simulink model "HDVSim_Hybrid_Truck"  (R2019b) (continued)
== system HDVSim_Hybrid_Truck/Electric motor ==
  note: Effective motor power
  note: Electric torque
  u_EM : Inport
  n_EM [rpm] : Inport  Port=2
  U_Bat [V] : Inport  Port=3
  Abs : Abs
  Abs1 : Abs
  Divide : Product  Inputs=*/
  Electric machine efficiency mapping : Lookup_n-D  BreakpointsForDimension1=em.efficiency.torque  BreakpointsForDimension2=em. efficiency.speed  Table=em.efficiency.characteristic_map  RndMeth=Simplest  InputSameDT=on
  Electric machine efficiency mapping2 : Lookup_n-D  BreakpointsForDimension1=em.efficiency.torque  BreakpointsForDimension2=em. efficiency.speed  Table=em.efficiency.characteristic_map  RndMeth=Simplest  InputSameDT=on
  Gain : Gain  Gain=2*pi/60
  Machine current demand : Subsystem
  Machine torque demand : Subsystem
  Math Function : Math  Operator=reciprocal  SaturateOnIntegerOverflow=on
  Product1 : Product
  Product2 : Product  Inputs=3
  Saturation : Saturate  UpperLimit=Bat.Useable_capacity / Bat.Useable_range*10  LowerLimit=-Bat.Useable_capacity / Bat.Useable_range*10
  Saturation1 : Saturate  UpperLimit=inf  LowerLimit=1
  Speed-torrque characteristics : Lookup_n-D  NumberOfTableDimensions=1  BreakpointsForDimension1=em.speed  Table=em.trq  ExtrapMethod=Clip  RndMeth=Simplest  InputSameDT=on
  Switch : Switch
  M_EM [Nm] : Outport  VectorParamsAs1DForOutWhenUnconnected=off
  I_EM_current [A] : Outport  Port=2  VectorParamsAs1DForOutWhenUnconnected=off
  tag scope: M_EM=global, n_EM=global, u_EM=global
  wires:
  Speed-torrque characteristics/1 -> Product1/1  "M_EM_max"
  u_EM/1 -> Product1/2, [u_EM]
  U_Bat [V]/1 -> Divide/2, Machine torque demand/4(U_Bat [V]), Machine current demand/4(U_Bat [V])  "U_Bat [V]"
  Machine current demand/1(I_target [A]) -> Saturation/1
  Saturation/1 -> Machine torque demand/2(I_current [A])
  Machine torque demand/1(M_EM [Nm]) -> Abs/1, Product2/3, [M_EM], M_EM [Nm]/1, Switch/2
  Gain/1 -> Product2/2
  Divide/1 -> I_EM_current [A]/1
  Math Function/1 -> Switch/1
  Electric machine efficiency mapping/1 -> Switch/3, Math Function/1  "Eta_EM"
  Switch/1 -> Product2/1
  Product2/1 -> Divide/1
  Abs/1 -> Electric machine efficiency mapping/1
  Product1/1 -> Machine current demand/2(M_EM_target [Nm]), Abs1/1  "M_EM_soll"
  n_EM [rpm]/1 -> Electric machine efficiency mapping2/2, Saturation1/1, Electric machine efficiency mapping/2, Machine torque demand/1(n_EM [rpm]), Machine current demand/1("n_EM "), [n_EM], Speed-torrque characteristics/1  "n_EM [1/min]"
  Abs1/1 -> Electric machine efficiency mapping2/1
  Electric machine efficiency mapping2/1 -> Machine current demand/3(Eff_EM), Machine torque demand/3(Eff_EM)  "ETA_EM"
  Saturation1/1 -> Gain/1

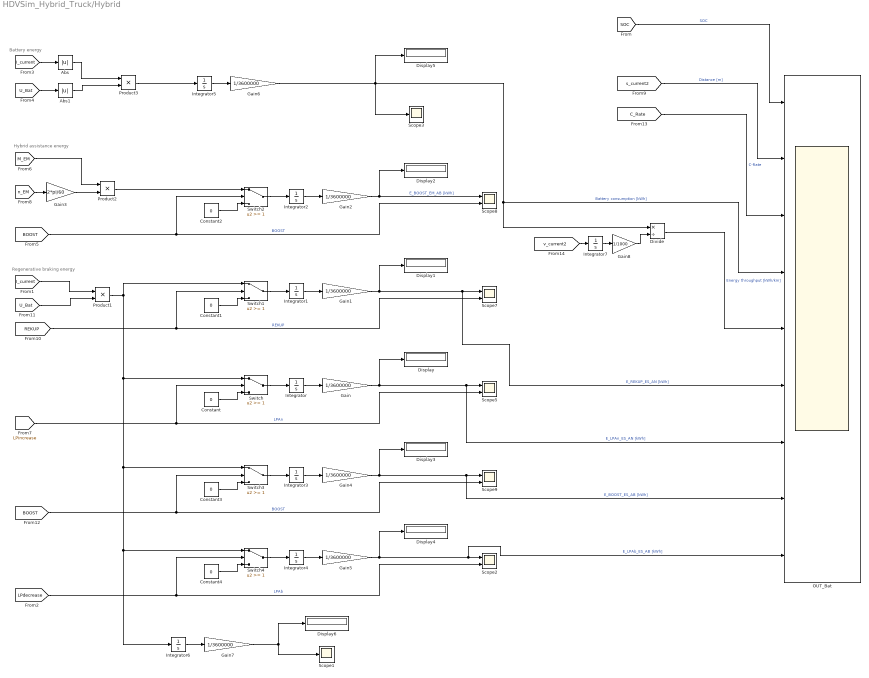
== system HDVSim_Hybrid_Truck/Hybrid ==
  note: Regenerative braking energy
  note: Hybrid assistance energy
  note: Battery energy
  Abs : Abs
  Abs1 : Abs
  Constant{,1..4} (5 alike) : Constant  Value=0
  Display{,1..6} (7 alike) : Display
  Divide : Product  Inputs=*/
  Gain{,1..2,4..7} (7 alike) : Gain  Gain=1/3600000
  Gain3 : Gain  Gain=2*pi/60
  Gain8 : Gain  Gain=1/1000
  Integrator{,1..6} (7 alike) : Integrator
  Integrator7 : Integrator  InitialCondition=0.00000001
  OUT_Bat : Scope  NumInputPorts=9
  Product1 : Product
  Product2 : Product
  Product3 : Product
  Scope1 : Scope
  Scope{2,5,7..9} (5 alike) : Scope  NumInputPorts=2
  Scope3 : Scope
  Switch{,1..4} (5 alike) : Switch  Threshold=1
  wires:
  Product1/1 -> Switch1/1, Switch/1, Switch3/1, Integrator6/1, Switch4/1
  Gain5/1 -> OUT_Bat/9, Scope2/1, Display4/1  "E_LPAb_ES_AB [kWh]"
  Constant4/1 -> Switch4/3
  Integrator4/1 -> Gain5/1
  Switch4/1 -> Integrator4/1
  [LPdecrease] -> Scope2/2, Switch4/2  "LPAb"
  Gain4/1 -> OUT_Bat/8, Scope9/1, Display3/1  "E_BOOST_ES_AB [kWh]"
  Constant3/1 -> Switch3/3
  Integrator3/1 -> Gain4/1
  Switch3/1 -> Integrator3/1
  [BOOST] -> Scope9/2, Switch3/2  "BOOST"
  Gain3/1 -> Product2/2
  [n_EM] -> Gain3/1
  Gain2/1 -> Scope8/1, Display2/1  "E_BOOST_EM_AB [kWh]"
  Constant2/1 -> Switch2/3
  Integrator2/1 -> Gain2/1
  Switch2/1 -> Integrator2/1
  [BOOST] -> Scope8/2, Switch2/2  "BOOST"
  Product2/1 -> Switch2/1
  Gain1/1 -> OUT_Bat/6, Scope7/1, Display1/1  "E_REKUP_ES_AN [kWh]"
  Constant1/1 -> Switch1/3
  Integrator1/1 -> Gain1/1
  Switch1/1 -> Integrator1/1
  [REKUP] -> Scope7/2, Switch1/2  "REKUP"
  [U_Bat] -> Product1/2
  [I_current] -> Product1/1
  Gain/1 -> OUT_Bat/7, Scope5/1, Display/1  "E_LPAn_ES_AN [kWh]"
  Constant/1 -> Switch/3
  Integrator/1 -> Gain/1
  Switch/1 -> Integrator/1
  [LPincrease] -> Scope5/2, Switch/2  "LPAn"
  [M_EM] -> Product2/1
  [SOC] -> OUT_Bat/1  "SOC"
  Gain7/1 -> Display6/1, Scope1/1
  Integrator6/1 -> Gain7/1
  [U_Bat] -> Abs1/1
  [I_current] -> Abs/1
  Product3/1 -> Integrator5/1
  Integrator5/1 -> Gain6/1
  Abs/1 -> Product3/1
  Abs1/1 -> Product3/2
  [s_current2] -> OUT_Bat/2  "Distance [m]"
  [C_Rate] -> OUT_Bat/3  "C-Rate"
  Divide/1 -> OUT_Bat/5  "Energy throughput [kWh/km]"
  [v_current2] -> Integrator7/1
  Integrator7/1 -> Gain8/1
  Gain8/1 -> Divide/2
  Gain6/1 -> Scope3/1, OUT_Bat/4, Divide/1, Display5/1  "Battery consumption [kWh]"

== system HDVSim_Hybrid_Truck/ICE engine ==
  note: Engine loading
  note: Consumption calculations
  u_ICE : Inport
  n_ICE [rpm] : Inport  Port=2
  Additional consumptions : Subsystem
  Consumption : Subsystem
  Drag torque : Lookup  InputValues=engine.drag_torque.speed  Table=engine.drag_torque.trq  LookUpMeth=Interpolation-Use End Values
  Full load curve : Lookup  InputValues=engine.full_load.speed  Table=engine.full_load.trq  LookUpMeth=Interpolation-Use End Values
  MinMax : MinMax  Inputs=2
  Product1 : Product
  Sum : Sum  Inputs=|+-
  Sum1 : Sum  Inputs=|++
  maximum engine torque due to tire slip : Subsystem
  M_ICE [Nm] : Outport  VectorParamsAs1DForOutWhenUnconnected=off
  tag scope: M_mot=global, n_mot=global
  wires:
  n_ICE [rpm]/1 -> Additional consumptions/2(n_clutch_en [rpm]), Consumption/2(n [rpm]), [n_mot], Drag torque/1, Full load curve/1
  Sum/1 -> Product1/2
  Product1/1 -> Sum1/1
  Drag torque/1 -> Sum1/2, Sum/2  "M_Drag"
  Additional consumptions/1(M_ICE_aftr_aux [Nm]) -> M_ICE [Nm]/1
  u_ICE/1 -> Product1/1
  Full load curve/1 -> MinMax/2  "M_ICE_max"
  Sum1/1 -> [M_mot], Additional consumptions/1(M_ICE_bfor_aux [Nm]), Consumption/1(M [Nm])
  maximum engine torque due to tire slip/1(M_mot_max_traction [Nm]) -> MinMax/1
  MinMax/1 -> Sum/1

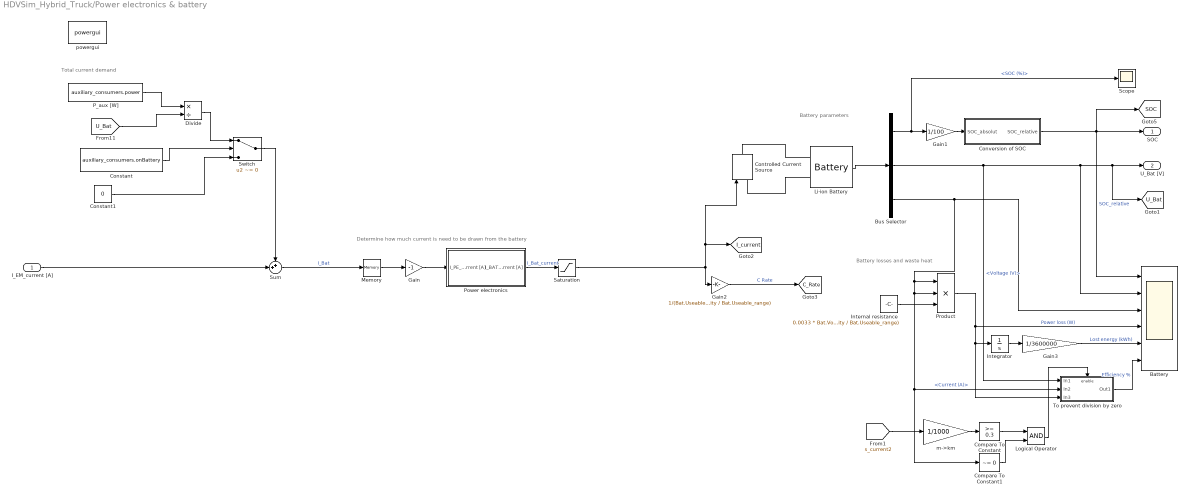
== system HDVSim_Hybrid_Truck/Power electronics & battery ==
  note: Battery parameters
  note: Battery losses and waste heat
  note: Total current demand
  note: Determine how much current is need to be drawn from the battery
  I_EM_current [A] : Inport
  Battery : Scope  NumInputPorts=6
  Bus Selector : BusSelector  OutputSignals=SOC (%),Voltage (V),Current (A)
  Compare To Constant : simulink/Logic and Bit Operations/Compare To Constant  relop=>=  const=0.3  OutDataTypeStr=uint8  ZeroCross=off
  Compare To Constant1 : simulink/Logic and Bit Operations/Compare To Constant  relop=~=  const=0  OutDataTypeStr=uint8  ZeroCross=off
  Constant : Constant  Value=auxiliary_consumers.onBattery
  Constant1 : Constant  Value=0
  Controlled Current Source : powerlib/Electrical Sources/Controlled Current Source  Initialize=off  Source_Type=DC  Amplitude=0  Phase=0  Frequency=0  Measurements=None
      settings: SourceProductName=Simscape Electrical
  Conversion of SOC : Subsystem
  Divide : Product  Inputs=*/
  Gain : Gain  Gain=-1
  Gain1 : Gain  Gain=1/100
  Gain2 : Gain  Gain=1/(Bat.Useable_capacity / Bat.Useable_range)
  Gain3 : Gain  Gain=1/3600000
  Integrator : Integrator
  Internal resistance : Constant  Value=0.0033 * Bat.Voltage / (Bat.Useable_capacity / Bat.Useable_range)
  Li-ion Battery : electricdrivelib/Extra Sources/Battery  BatType=Lithium-Ion  ShowTempParam=off  ThermalPreset=no  ShowAgeParam=off  NomV=Bat.Voltage  NomQ=Bat.Useable_capacity / Bat.Useable_range  SOC=(Bat.SOC_start *Bat.Useable_range+(0.5-Bat.Useable_range/2))* 100  Batt_Tr=30  PresetModel=off  MaxQ=Bat.Useable_capacity / Bat.Useable_range *8077/7500  MinV=487.5  FullV=Bat.Voltage * 1.178  Dis_rate=Bat.Useable_capacity / Bat.Useable_range * 1  R=0.0033 * Bat.Voltage / (Bat.Useable_capacity / Bat.Useable_range)  Normal_OP=Bat.Useable_capacity / Bat.Useable_range * 0.962  expZone="[Bat.Voltage*4339/4000     Bat.Useable_capacity / Bat.Useable_range/5]"  current=[5 25 50 250]  Units=Ampere-hour  Tci=20  NomT=20  T2=-30  MaxQ2=Bat.Useable_capacity / Bat.Useable_range *8077/7500  FullV2=Bat.Voltage * 1.178  Normal_OP2=487.5  expZone2="[Bat.Voltage*4339/4000     Bat.Useable_capacity / Bat.Useable_range/5]"  Rca=0.6  ti=1000  Dp=0  Neq0=0  Tsim=1e6  Ta1=25  Qeol=5.4*0.9  Reol=0.013333*1.2  Char_age=[2.3478, 3]  Dis_age=[2.3478, 10]  N1=1500  N2=10500  N3=1000  N4=1400  Ta2=45  N5=950
      settings: SourceProductName=Simscape Electrical
  Logical Operator : Logic  AllPortsSameDT=off  OutDataTypeStr=boolean
  Memory : Memory
  P_aux [W] : Constant  Value=auxiliary_consumers.power
  Power electronics : Subsystem
  Product : Product  Inputs=3
  Saturation : Saturate  UpperLimit=450  LowerLimit=-450
  Scope : Scope
  Sum : Sum  Inputs=++|
  Switch : Switch  Criteria=u2 ~= 0
  To prevent division by zero : Subsystem
  m->km : Gain  Gain=1/1000
  powergui : powerlib/powergui  Priority=1  SimulationMode=Discrete  SampleTime=sample_time  frequency=60  Iterations=50  frequencyindice=0  Pbase=100e6  ErrMax=1e-4  UnitsV=kV  UnitsW=MW  buscounter=0  FunctionMessages=off  echomessages=off  EnableUseOfTLC=off  CurrentSourceSwitches=off  DisableSnubberDevices=off  DisableRonSwitches=off  DisableVfSwitches=off  DisplayEquations=off  AutomaticDiscreteSolvers=off  SolverType=Tustin  Interpol=off  ExternalGateDelay=off  methode=off  Ts=100  x0status=blocks  SPID=off  SwTol=0  HookPort=off  NonlinearTolerance=1e-4  nMaxIteration=100  ContinueOnMaxIteration=off  RestoreLinks=warning  ResistiveCurrentMeasurement=off  Frange=[0:2:500]  Ylog=off  Xlog=on  ShowGrid=off  save=off  variable=ZData  structure=0  ZoomFFT=off  StartTime=0.0  cycles=1  DisplayStyle=1  fundamental=60  FreqAxis=off  MaxFrequency=1000  frequencyindicesteady=1  RmsSteady=1  display=off
      settings: SourceProductName=Simscape Electrical
  SOC : Outport  VectorParamsAs1DForOutWhenUnconnected=off
  U_Bat [V] : Outport  Port=2  VectorParamsAs1DForOutWhenUnconnected=off
  tag scope: C_Rate=global, I_current=global, SOC=global, U_Bat=global
  wires:
  Li-ion Battery/1 -> Bus Selector/1
  Bus Selector/1 -> Scope/1, Gain1/1  "<SOC (%)>"
  Bus Selector/2 -> To prevent division by zero/1(In1), U_Bat [V]/1, [U_Bat], Battery/2  "<Voltage (V)>"
  Gain1/1 -> Conversion of SOC/1(SOC_absolut)
  Conversion of SOC/1(SOC_relative) -> SOC/1, [SOC], Battery/1  "SOC_relative"
  I_EM_current [A]/1 -> Sum/2
  Power electronics/1(I_BAT_current [A]) -> Saturation/1  "I_Bat_current"
  Gain2/1 -> [C_Rate]  "C Rate"
  [U_Bat] -> Divide/2
  P_aux [W]/1 -> Divide/1
  Constant/1 -> Switch/2
  Divide/1 -> Switch/1
  Constant1/1 -> Switch/3
  Switch/1 -> Sum/1
  Bus Selector/3 -> Product/1, To prevent division by zero/2(In2), Compare To Constant1/1, Product/2, Battery/3  "<Current (A)>"
  Internal resistance/1 -> Product/3
  Product/1 -> Battery/4, To prevent division by zero/3(In3), Integrator/1  "Power loss (W)"
  Integrator/1 -> Gain3/1
  Gain3/1 -> Battery/5  "Lost energy (kWh)"
  [s_current2] -> m->km/1
  m->km/1 -> Compare To Constant/1
  To prevent division by zero/1(Out1) -> Battery/6  "Efficiency %"
  Compare To Constant/1 -> Logical Operator/1
  Logical Operator/1 -> To prevent division by zero/Enable
  Compare To Constant1/1 -> Logical Operator/2
  Saturation/1 -> Controlled Current Source/1, [I_current], Gain2/1
  Gain/1 -> Power electronics/1(I_PE_current [A])
  Sum/1 -> Memory/1  "I_Bat"
  Memory/1 -> Gain/1
  connections:
  Li-ion Battery/LConn1 -- Controlled Current Source/RConn1
  Li-ion Battery/LConn2 -- Controlled Current Source/LConn1

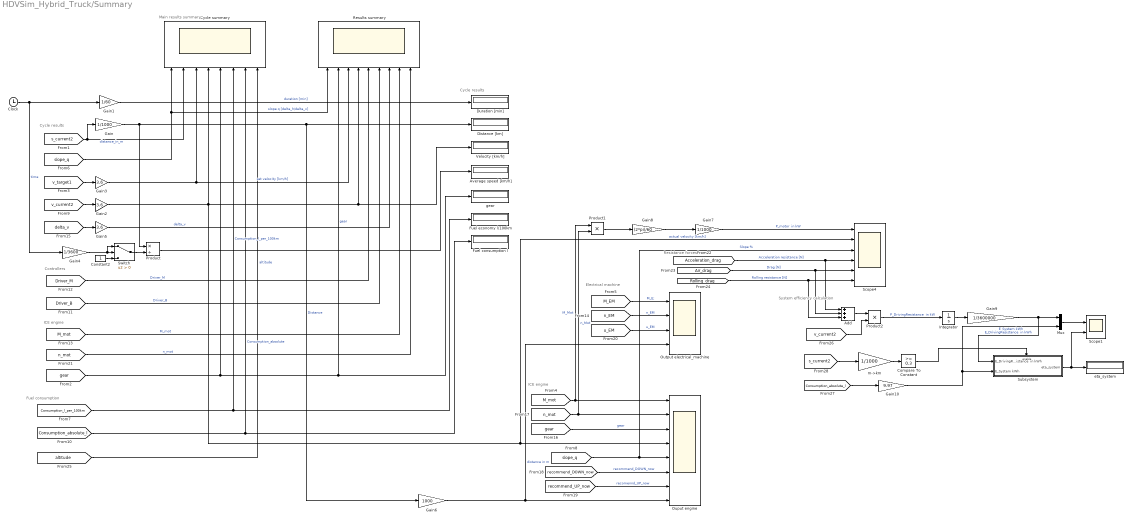
== system HDVSim_Hybrid_Truck/Summary ==
  note: Resistance forces
  note: Electrical machine
  note: ICE engine
  note: Main results summary
  note: Cycle results
  note: Fuel consumption
  note: ICE engine
  note: Controllers
  note: Cycle results
  note: System efficiency calculation
  Add : Sum  Inputs=+++
  Average speed [km/h] : Display
  Clock : Clock
  Compare To Constant : simulink/Logic and Bit Operations/Compare To Constant  relop=>=  const=0.3  OutDataTypeStr=uint8  ZeroCross=off
  Constant2 : Constant
  Cycle summary : Scope  NumInputPorts=8
  Distance [km] : Display
  Duration [min] : Display
  Fuel consumption l : Display
  Fuel economy l/100km : Display
  Gain : Gain  Gain=1/1000
  Gain1 : Gain  Gain=1/60
  Gain10 : Gain  Gain=9.97
  Gain2 : Gain  Gain=3.6
  Gain3 : Gain  Gain=3.6
  Gain4 : Gain  Gain=1/3600
  Gain5 : Gain  Gain=3.6
  Gain6 : Gain  Gain=1000
  Gain7 : Gain  Gain=1/1000
  Gain8 : Gain  Gain=(2*pi)/60
  Gain9 : Gain  Gain=1/3600000
  Integrator : Integrator
  Mux : Mux  Inputs=2
  Ouput engine : Scope  NumInputPorts=8
  Output electrical_machine : Scope  NumInputPorts=4
  Product : Product  Inputs=*/  RndMeth=Zero
  Product1 : Product
  Product2 : Product
  Results summary : Scope  NumInputPorts=9
  Scope1 : Scope  NumInputPorts=2
  Scope4 : Scope  NumInputPorts=6
  Subsystem : Subsystem
  Switch : Switch  Criteria=u2 > Threshold
  Velocity [km/h] : Display
  eta_system : Display
  gear : Display
  m->km : Gain  Gain=1/1000
  wires:
  [v_current2] -> Gain2/1
  [s_current2] -> Cycle summary/2, Gain/1  "distance_in_m"
  Gain/1 -> Distance [km]/1, Gain6/1, Product/1  "Distance"
  Clock/1 -> Gain1/1, Gain4/1  "time"
  Gain1/1 -> Duration [min]/1  "duration [min]"
  Gain2/1 -> Ouput engine/4, Scope4/2, Results summary/4, Velocity [km/h]/1, Cycle summary/4  "actual velocity [km/h]"
  [gear] -> gear/1, Results summary/2, Cycle summary/5  "gear"
  [v_target1] -> Gain3/1
  [slope_q] -> Results summary/1, Cycle summary/1  "slope q [delta_h/delta_x]"
  [Consumption_l_per_100km] -> Cycle summary/6, Fuel economy l/100km/1  "Consumption_l_per_100km"
  Gain3/1 -> Cycle summary/3, Results summary/3  "set velocity [km/h]"
  Product/1 -> Average speed [km/h]/1
  [Consumption_absolute_l] -> Cycle summary/7, Fuel consumption l/1  "Consumption_absolute"
  [Driver_M] -> Results summary/5  "Driver_M"
  [Driver_B] -> Results summary/6  "Driver_B"
  [M_mot] -> Results summary/8  "M_mot"
  [delta_v] -> Gain5/1
  [M_mot] -> Ouput engine/1, Product1/1  "M_Mot"
  [n_mot] -> Ouput engine/2, Product1/2  "n_Mot"
  [gear] -> Ouput engine/3  "gear"
  [recommend_DOWN_now] -> Ouput engine/6  "recommend_DOWN_now"
  [recommend_UP_now] -> Ouput engine/7  "recomennd_UP_now"
  [slope_q] -> Ouput engine/5, Scope4/3  "Slope %"
  Gain5/1 -> Results summary/7  "delta_v"
  [n_mot] -> Results summary/9  "n_mot"
  [M_EM] -> Output electrical_machine/1  "M_E;"
  [n_EM] -> Output electrical_machine/2  "n_EM"
  [u_EM] -> Output electrical_machine/3  "u_EM"
  Product1/1 -> Gain8/1
  Gain7/1 -> Scope4/1  "P_motor  in kW"
  Gain4/1 -> Switch/1, Switch/2
  Constant2/1 -> Switch/3
  Switch/1 -> Product/2
  [altitude] -> Cycle summary/8  "altitude"
  Gain6/1 -> Output electrical_machine/4, Ouput engine/8  "distance in m"
  Gain8/1 -> Gain7/1
  Subsystem/1(eta_system) -> eta_system/1, Scope1/2
  Mux/1 -> Scope1/1
  [v_current2] -> Product2/2
  [s_current2] -> m->km/1
  m->km/1 -> Compare To Constant/1
  Gain10/1 -> Mux/2, Subsystem/2(E_System kWh)  "E_System kWh"
  Gain9/1 -> Subsystem/1(E_DrivingResistance  in kWh), Mux/1  "E_DrivingResistance  in kWh"
  Integrator/1 -> Gain9/1
  [Consumption_absolute_l] -> Gain10/1
  Add/1 -> Product2/1
  Product2/1 -> Integrator/1  "P_DrivingResistance  in kW"
  Compare To Constant/1 -> Subsystem/Enable
  [Acceleration_drag] -> Add/1, Scope4/4  "Acceleration resistance [N]"
  [Air_drag] -> Add/2, Scope4/5  "Drag [N]"
  [Rolling_drag] -> Add/3, Scope4/6  "Rolling resistance [N]"

== system HDVSim_Hybrid_Truck/Tires ==
  M_tire [Nm] : Inport
  v_current [m/s] : Inport  Port=2
  F=M/r : Product  Inputs=*/
  Tire radius : Constant  Value=tires.radius
  "phi. -> n[1/min]" : Gain  Gain=60/2/pi
  phi. = v/r : Product  Inputs=/*
  n_tire [rpm] : Outport  VectorParamsAs1DForOutWhenUnconnected=off
  F_x [N] : Outport  Port=2  VectorParamsAs1DForOutWhenUnconnected=off
  wires:
  phi. = v/r/1 -> "phi. -> n[1/min]"/1
  "phi. -> n[1/min]"/1 -> n_tire [rpm]/1
  Tire radius/1 -> F=M/r/2, phi. = v/r/1
  v_current [m/s]/1 -> phi. = v/r/2
  M_tire [Nm]/1 -> F=M/r/1
  F=M/r/1 -> F_x [N]/1

== system HDVSim_Hybrid_Truck/Transmission ==
  note: Transmission torque multiplication through the different gears
  M_EM [Nm] : Inport
  M_clutch_dis [Nm] : Inport  Port=2
  n_Gearbox [rpm] : Inport  Port=3
  alpha : Inport  Port=4
  Determine when shifting occurs : Subsystem  TreatAsAtomicUnit=on
  Memory1 : Memory
  Number of gears : Constant  Value=length(transmission.ratios)
  Saturation : Saturate  UpperLimit=4000  LowerLimit=0
  Shifting strategy1 : Stateflow chart  TreatAsAtomicUnit=on
  Sum : Sum
  Torque flow through the transmission : Subsystem
  ZKU : Subsystem
  init_gear : Constant  Value=transmission.init_gear
  n_clutch_dis [rpm] : Outport  VectorParamsAs1DForOutWhenUnconnected=off
  M_Gearbox [Nm] : Outport  Port=2  VectorParamsAs1DForOutWhenUnconnected=off
  tag scope: gear=global, i_gearbox=global, recommend_DOWN_now=global, recommend_UP_now=global
  wires:
  n_Gearbox [rpm]/1 -> Torque flow through the transmission/2(n_gearbox_out [1/min]), Determine when shifting occurs/2(n_Gear_out [1/min])
  Torque flow through the transmission/2(M_gearbox_out [Nm]) -> [M_Gear_out], M_Gearbox [Nm]/1
  Torque flow through the transmission/1(n_gearbox_in [1/min]) -> Saturation/1
  init_gear/1 -> Shifting strategy1/1(init_gear)
  Determine when shifting occurs/27(check_torque_reseve_2lower) -> Shifting strategy1/29(check_torque_reserve_2lower)
  Determine when shifting occurs/26(check_torque_reseve_2higher) -> Shifting strategy1/28(check_torque_reserve_2higher)
  Determine when shifting occurs/1(reccommend_down_2higher) -> Shifting strategy1/3(recommend_down_2higher)
  Determine when shifting occurs/2(reccommend_down_1higher) -> Shifting strategy1/4(recommend_down_1higher)
  Determine when shifting occurs/3(reccommend_down_now) -> Shifting strategy1/5(recommend_down_now), [recommend_DOWN_now]
  Determine when shifting occurs/4(reccommend_down_1lower) -> Shifting strategy1/6(recommend_down_1lower)
  Determine when shifting occurs/5(reccommend_down_2lower) -> Shifting strategy1/7(recommend_down_2llower)
  Determine when shifting occurs/6(reccommend_up_2higher) -> Shifting strategy1/8(recommend_up_2higher)
  Determine when shifting occurs/7(reccommend_up_1higher) -> Shifting strategy1/9(recommend_up_1higher)
  Determine when shifting occurs/8(reccommend_up_now) -> Shifting strategy1/10(recommend_up_now), [recommend_UP_now]
  Determine when shifting occurs/9(reccommend_up_1lower) -> Shifting strategy1/11(recommend_up_1lower)
  Determine when shifting occurs/10(reccommend_up_2lower) -> Shifting strategy1/12(recommend_up_2lower)
  Determine when shifting occurs/11(check_n_min_2higher) -> Shifting strategy1/13(check_n_min_2higher)
  Determine when shifting occurs/12(check_n_min_1higher) -> Shifting strategy1/14(check_n_min_1higher)
  Determine when shifting occurs/13(check_n_min_now) -> Shifting strategy1/15(check_n_min_now)
  Determine when shifting occurs/14(check_n_min_1lower) -> Shifting strategy1/16(check_n_min_1lower)
  Determine when shifting occurs/15(check_n_min_2lower) -> Shifting strategy1/17(check_n_min_2lower)
  Determine when shifting occurs/16(check_n_max_2higher) -> Shifting strategy1/18(check_n_max_2higher)
  Determine when shifting occurs/17(check_n_max_1higher) -> Shifting strategy1/19(check_n_max_1higher)
  Determine when shifting occurs/18(check_n_max_now) -> Shifting strategy1/20(check_n_max_now)
  Determine when shifting occurs/19(check_n_max_1lower) -> Shifting strategy1/21(check_n_max_1lower)
  Determine when shifting occurs/20(check_n_max_2lower) -> Shifting strategy1/22(check_n_max_2lower)
  Determine when shifting occurs/21(check_M_max_2higher) -> Shifting strategy1/23(check_M_max_2higher)  "check_M_max_2higher"
  Determine when shifting occurs/22(check_M_max_1higher) -> Shifting strategy1/24(check_M_max_1higher)  "check_M_max_1higher"
  Determine when shifting occurs/23(check_M_max_now) -> Shifting strategy1/25(check_M_max_now)  "check_M_max_now"
  Determine when shifting occurs/24(check_M_max_1lower) -> Shifting strategy1/26(check_M_max_1lower)  "check_M_max_1lower"
  Determine when shifting occurs/25(check_M_max_2lower) -> Shifting strategy1/27(check_M_max_2lower)  "check_M_max_2lower"
  Memory1/1 -> [Gear], ZKU/1(Gear), Torque flow through the transmission/3(Gear), [gear]
  M_clutch_dis [Nm]/1 -> Sum/2
  Sum/1 -> Torque flow through the transmission/1(M_gearbox_in [Nm])  "M_gearbox_in [Nm]"
  Torque flow through the transmission/3(i_gearbox) -> [i_gearbox]
  Saturation/1 -> n_clutch_dis [rpm]/1
  M_EM [Nm]/1 -> Sum/1, Determine when shifting occurs/4(M_EM [Nm])
  Number of gears/1 -> Shifting strategy1/2(z)
  alpha/1 -> Determine when shifting occurs/5(alpha)
  [gear] -> Memory1/1
  Shifting strategy1/1(gear) -> [gear]
  [Gear] -> Determine when shifting occurs/1(Gear)
  [M_Gear_out] -> Determine when shifting occurs/3(M_Gear_out [Nm])

== system HDVSim_Hybrid_Truck/Vehicle ==
  note: Net tractive force applied on the vehicle
  note: Vehicle acceleration
  F_x [N] : Inport
  u_KB : Inport  Port=2
  alpha [rad] : Inport  Port=3
  Add : Sum  Inputs=-+-
  Breaking force [N] : Product  RndMeth=Zero
  Cargo weight : Constant  Value=vehicle.payload
  Driving resistances without acceleration : Subsystem
  F_brake_max : Constant  Value=vehicle.F_brake_max
  Integrator : Integrator
  Integrator1 : Integrator  InitialCondition=cycle.speed_init  LowerSaturationLimit=0
  Product : Product  Inputs=3
  Product2 : Product  Inputs=*/  RndMeth=Zero
  Product3 : Product  Inputs=**  RndMeth=Zero
  Saturation : Saturate  UpperLimit=15  LowerLimit=-15
  Sum1 : Sum  Inputs=|++
  Sum2 : Sum  Inputs=|++
  Vehicle weight : Constant  Value=vehicle.mass
  v_current [m/s] : Outport  VectorParamsAs1DForOutWhenUnconnected=off
  s_current [m] : Outport  Port=2  VectorParamsAs1DForOutWhenUnconnected=off
  tag scope: Acceleration_drag=global, s_current2=global, v_current2=global
  wires:
  alpha [rad]/1 -> Driving resistances without acceleration/1(alpha [rad])
  Add/1 -> Product2/1
  Driving resistances without acceleration/1(F_W [N]) -> Add/3
  F_x [N]/1 -> Add/2  "F_x"
  F_brake_max/1 -> Breaking force [N]/2
  Integrator/1 -> [s_current2], s_current [m]/1  "s_ist"
  Cargo weight/1 -> Sum1/2, Sum2/2
  Sum1/1 -> Product2/2
  Vehicle weight/1 -> Sum2/1, Product3/2
  Product3/1 -> Sum1/1
  Integrator1/1 -> Driving resistances without acceleration/2(v [m/s]), v_current [m/s]/1, Integrator/1, [v_current2]  "v_ist"
  [lamda] -> Product/1, Product3/1
  u_KB/1 -> Breaking force [N]/1
  Breaking force [N]/1 -> Add/1  "F_Brems"
  Product2/1 -> Saturation/1, Product/3  "a"
  Product/1 -> [Acceleration_drag]
  Sum2/1 -> Product/2  "m_total"
  Saturation/1 -> Integrator1/1

== system HDVSim_Hybrid_Truck/Active cruise control/Braking from upper speed limit ==
  v_target [m/s] : Inport
  Add3 : Sum  Inputs=+-
  Constant1 : Constant  Value=90/3.6
  Constant10 : Constant  Value=preditiveCruiseControl
  Constant6 : Constant  Value=v_PPC_delta
  Constant7 : Constant  Value=0
  Constant8 : Constant  Value=0
  Saturation Dynamic : simulink/Discontinuities/Saturation Dynamic  OutMin=[]  OutMax=[]  OutDataTypeStr=Inherit: Same as second input  OutDataType=fixdt(1, 16)  OutScaling=2^-10  DoSatur=off
  Switch3 : Switch  Criteria=u2 > Threshold
  Out1 : Outport  VectorParamsAs1DForOutWhenUnconnected=off
  wires:
  Switch3/1 -> Out1/1
  Constant6/1 -> Saturation Dynamic/2
  Constant1/1 -> Add3/1
  Constant7/1 -> Switch3/3
  Add3/1 -> Saturation Dynamic/1
  Constant8/1 -> Saturation Dynamic/3
  Constant10/1 -> Switch3/2
  v_target [m/s]/1 -> Add3/2
  Saturation Dynamic/1 -> Switch3/1

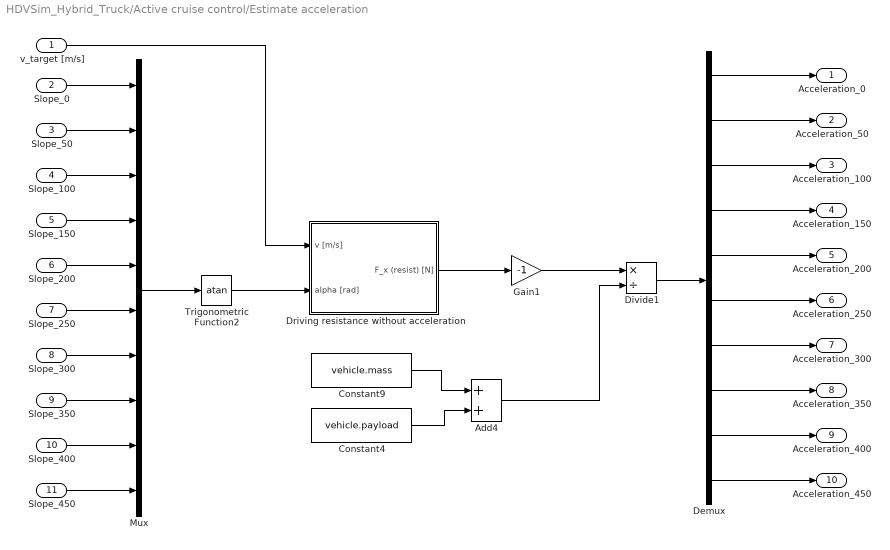
== system HDVSim_Hybrid_Truck/Active cruise control/Estimate acceleration ==
  v_target [m/s] : Inport
  Slope_0 : Inport  Port=2
  Slope_50 : Inport  Port=3
  Slope_100 : Inport  Port=4
  Slope_150 : Inport  Port=5
  Slope_200 : Inport  Port=6
  "Slope_250 " : Inport  Port=7
  Slope_300 : Inport  Port=8
  Slope_350 : Inport  Port=9
  Slope_400 : Inport  Port=10
  Slope_450 : Inport  Port=11
  Add4 : Sum
  Constant4 : Constant  Value=vehicle.payload
  Constant9 : Constant  Value=vehicle.mass
  Demux : Demux  Outputs=10
  Divide1 : Product  Inputs=*/
  Driving resistance without acceleration : Subsystem
  Gain1 : Gain  Gain=-1
  Mux : Mux  Inputs=10
  Trigonometric Function2 : Trigonometry  Operator=atan
  Acceleration_0 : Outport  VectorParamsAs1DForOutWhenUnconnected=off
  Acceleration_50 : Outport  Port=2  VectorParamsAs1DForOutWhenUnconnected=off
  Acceleration_100 : Outport  Port=3  VectorParamsAs1DForOutWhenUnconnected=off
  Acceleration_150 : Outport  Port=4  VectorParamsAs1DForOutWhenUnconnected=off
  Acceleration_200 : Outport  Port=5  VectorParamsAs1DForOutWhenUnconnected=off
  Acceleration_250 : Outport  Port=6  VectorParamsAs1DForOutWhenUnconnected=off
  Acceleration_300 : Outport  Port=7  VectorParamsAs1DForOutWhenUnconnected=off
  Acceleration_350 : Outport  Port=8  VectorParamsAs1DForOutWhenUnconnected=off
  Acceleration_400 : Outport  Port=9  VectorParamsAs1DForOutWhenUnconnected=off
  Acceleration_450 : Outport  Port=10  VectorParamsAs1DForOutWhenUnconnected=off
  wires:
  v_target [m/s]/1 -> Driving resistance without acceleration/1(v [m/s])
  Trigonometric Function2/1 -> Driving resistance without acceleration/2(alpha [rad])
  Constant9/1 -> Add4/1
  Constant4/1 -> Add4/2
  Driving resistance without acceleration/1(F_x (resist) [N]) -> Gain1/1
  Add4/1 -> Divide1/2
  Gain1/1 -> Divide1/1
  Mux/1 -> Trigonometric Function2/1
  Divide1/1 -> Demux/1
  Demux/1 -> Acceleration_0/1
  Slope_0/1 -> Mux/1
  Slope_50/1 -> Mux/2
  Slope_100/1 -> Mux/3
  Slope_150/1 -> Mux/4
  Slope_200/1 -> Mux/5
  "Slope_250 "/1 -> Mux/6
  Slope_300/1 -> Mux/7
  Slope_350/1 -> Mux/8
  Slope_400/1 -> Mux/9
  Slope_450/1 -> Mux/10
  Demux/2 -> Acceleration_50/1
  Demux/3 -> Acceleration_100/1
  Demux/4 -> Acceleration_150/1
  Demux/5 -> Acceleration_200/1
  Demux/6 -> Acceleration_250/1
  Demux/7 -> Acceleration_300/1
  Demux/8 -> Acceleration_350/1
  Demux/9 -> Acceleration_400/1
  Demux/10 -> Acceleration_450/1
